simulink model "nonlinear_double_track_model"  (R2024b)
settings: Solver=VariableStepAuto  StartTime=0.0  StopTime=5  FixedStep=0.01  RelTol=1e-4
undefined symbols (not defined in the file or in its folder): a, b, balance_bar_ratio_front, brake_pedal_motion_ratio, cg_height, disc_radius_front, disc_radius_rear, front_mass_distribution, g, gear_ratio, Jeq, Ki_yaw, Kp_yaw, mass_total, max_pedal_force, mc_diameter_front, mc_diameter_rear, num_pistons_front, num_pistons_rear, pad_friction_front, pad_friction_rear, pad_height_front, pad_height_rear, piston_radius_front, piston_radius_rear, static_toe_front, static_toe_rear, tire_loaded_radius, tire_mu, tire_mu_correction_factor, tlltd, toe_deflection_rear, track_width_front, track_width_rear, wheelbase, yaw_polar_inertia
libraries: simulink_extras x12, checkpacklib x1, slpidlib x1
(part 6 of 6)

== matlab function nonlinear_double_track_model/Vehicle Plant Model/Slip Ratio Calculation/Slip Ratio Calculation ==
  data: sl_lf output; sl_rf output; sl_lr output; sl_rr output; w_fl input; w_fr input; w_rl input; w_rr input; vs_fl input; vs_fr input; vs_rl input; vs_rr input; effective_radius input
%{

Slip Ratio:
We should expect SL to be (+) when the wheel is spinning faster than the
tire surface velocity, resulting in forward acceleration. SL should be (-)
for drag or braking forces, resuling in negative long acceleration.

%}
function [sl_lf, sl_rf, sl_lr, sl_rr] = fcn(w_fl, w_fr, w_rl, w_rr, vs_fl, vs_fr, vs_rl, vs_rr, effective_radius)

% Note: in calspan info N is in RPM. Here we use rad/s so we compare
% apples-to-apples. No difference.

%% Calculate slip angles
% Note: a better approach is to calculate RE as a function of V, Fz ->
% RE(V, Fz) for each wheel individually, and use a lookup table for RE
% derived from the tire test data
sl_lf = (w_fl * effective_radius / vs_fl) - 1;
sl_rf = (w_fr * effective_radius / vs_fr) - 1;
sl_lr = (w_rl * effective_radius / vs_rl) - 1;
sl_rr = (w_rr * effective_radius / vs_rr) - 1;

== matlab function nonlinear_double_track_model/Vehicle Plant Model/Mechanical Brake System/Brake Torque FL ==
  data: pressure input; brake_torque output; wheel_speed input; piston_radius input; num_pistons input; pad_height input; mu input; disc_radius input
function brake_torque = fcn(pressure, wheel_speed, piston_radius, num_pistons, pad_height, mu, disc_radius)

% caculate radius to center of pad
pad_center_radius = disc_radius - (pad_height / 2);

% calculate total piston area per caliper
piston_area = pi * (piston_radius)^2 * num_pistons;

% calculate caliper force
caliper_force = mu * pressure * piston_area;

% calculate brake torque
if (wheel_speed > 0)
    brake_torque = -caliper_force * pad_center_radius;
elseif (wheel_speed < 0)
    brake_torque = caliper_force * pad_center_radius;
else
    % Note: for speed = 0, the proper brake torque is equal & opposite to
    % the applied wheel torque. to do some day, but i dont care
    brake_torque = 0;
end

== matlab function nonlinear_double_track_model/Vehicle Plant Model/Mechanical Brake System/Brake Torque FL2 ==
  data: pressure input; brake_torque output; wheel_speed input; piston_radius input; num_pistons input; pad_height input; mu input; disc_radius input
function brake_torque = fcn(pressure, wheel_speed, piston_radius, num_pistons, pad_height, mu, disc_radius)

% caculate radius to center of pad
pad_center_radius = disc_radius - (pad_height / 2);

% calculate total piston area per caliper
piston_area = pi * (piston_radius)^2 * num_pistons;

% calculate caliper force
caliper_force = mu * pressure * piston_area;

% calculate brake torque
if (wheel_speed > 0)
    brake_torque = -caliper_force * pad_center_radius;
elseif (wheel_speed < 0)
    brake_torque = caliper_force * pad_center_radius;
else
    % Note: for speed = 0, the proper brake torque is equal & opposite to
    % the applied wheel torque. to do some day, but i dont care
    brake_torque = 0;
end

== matlab function nonlinear_double_track_model/Vehicle Plant Model/Mechanical Brake System/Brake Torque FL3 ==
  data: pressure input; brake_torque output; wheel_speed input; piston_radius input; num_pistons input; pad_height input; mu input; disc_radius input
function brake_torque = fcn(pressure, wheel_speed, piston_radius, num_pistons, pad_height, mu, disc_radius)

% caculate radius to center of pad
pad_center_radius = disc_radius - (pad_height / 2);

% calculate total piston area per caliper
piston_area = pi * (piston_radius)^2 * num_pistons;

% calculate caliper force
caliper_force = mu * pressure * piston_area;

% calculate brake torque
if (wheel_speed > 0)
    brake_torque = -caliper_force * pad_center_radius;
elseif (wheel_speed < 0)
    brake_torque = caliper_force * pad_center_radius;
else
    % Note: for speed = 0, the proper brake torque is equal & opposite to
    % the applied wheel torque. to do some day, but i dont care
    brake_torque = 0;
end

== matlab function nonlinear_double_track_model/Vehicle Plant Model/Aero Forces/MATLAB Function ==
  data: a input; downforce_front output; b input; downforce input; center_of_pressure input; downforce_rear output
function [downforce_front, downforce_rear] = fcn(a, b, downforce, center_of_pressure)

downforce_front = downforce * (center_of_pressure + b) / (a + b);
downforce_rear = downforce * (a - center_of_pressure) / (a + b);

== matlab function nonlinear_double_track_model/Vehicle Plant Model/Wheel Dynamics/Initial Velocity to Initial wheel speed ==
  data: v input; w output; r input
function w = fcn(v, r)

w = v/r;

== matlab function nonlinear_double_track_model/Vehicle Plant Model/Normal Force Calculation1/Normal Load Calculation ==
  data: total_mass input; fz_fl output; fz_fr output; fz_rl output; fz_rr output; g input; front_mass_distribution input; tlltd input; cg_height input; track_width_front input; track_width_rear input; wheelbase input; fy_vehicle input; fx_vehicle input; fz_total output; on_two_wheels output; front_downforce input; rear_downforce input
%{
    
Calculates the normal forces on each tire. It considers the static normal
load, long. load transfer, lateral load transfer using a distribution
constant, and checks for tires lifiting off the ground. It ensures the
total normal load is equal to the vehicle normal force. When multiple tires
are off the ground the load distribution in the grounded tires may be off.
Load transfer from Mx is not implemented.

Note: for aerodynamics add those after this block and not in it.

Inputs:
- total_mass: Total mass of the vehicle (kg)
- g: Gravitational acceleration (m/s^2)
- static_wd: Static weight distribution as [front, rear]
- tlltd: Total lateral load transfer distribution (percentage)
- longitudinal_transfer: Longitudinal load transfer (N)
- lateral_transfer: Lateral load transfer (N)
- fy_vehicle: total lateral force acting on vehicle CG (N)
- fx_vehicle: total longitudinal force acting on vehicle CG (N)
- front_downforce: downforce at front axle. This will be negative (N)
- rear_downforce: downforce at rear axle. This will be negative (N)

Force Directions
when looking from th top of the vehicle:
(+) fx_vehicle is forward
(+) fy_vehicle is to the left

%}

function [fz_fl, fz_fr, fz_rl, fz_rr, fz_total, on_two_wheels] = normal_loads(total_mass, g, front_mass_distribution, tlltd, cg_height, track_width_front, track_width_rear, wheelbase, fy_vehicle, fx_vehicle, front_downforce, rear_downforce)

%% Constants and initial calculations
total_normal_load = total_mass * g; % Total vertical load (N)
static_load_front = front_mass_distribution * total_normal_load;
static_load_rear = (1 - front_mass_distribution) * total_normal_load;
track_width = (track_width_front + track_width_rear) / 2;

%% Static normal loads  as [FL, FR, RL, RR]
Fz_static = [static_load_front / 2, static_load_front / 2, static_load_rear / 2, static_load_rear / 2];

%% Calculate lateral load transfer
% from left tires to right tires
lateral_transfer = (fy_vehicle * cg_height) / track_width;
Fz_transfer_lat = lateral_transfer .* [-tlltd, tlltd, -(1 - tlltd), (1 - tlltd)];


%% Calculate longitudinal load transfer
% from front tires to rear tires
longitudinal_transfer = (fx_vehicle * cg_height) / wheelbase;
Fz_transfer_long = longitudinal_transfer .* [-1/2, -1/2, 1/2, 1/2];

%% Calculate downforce contribution
Fz_downforce = [-front_downforce/2, -front_downforce/2, -rear_downforce/2, -rear_downforce/2];
total_downforce = sum(Fz_downforce);

% Combine static and transfer loads
fz = Fz_static + Fz_transfer_long + Fz_transfer_lat + Fz_downforce;

%% Clamp negative loads to zero
fz(fz < 0) = 0; % if a tire is off the ground, make it zero so tire model doesnt go crazy

%% Check if redistribution is necessary
% find the tires that are on the ground
grounded_tires = fz > 0;

% Redistribution is needed only if a tire is off the ground
if sum(grounded_tires) < 4

    % correct the other tire normal loads for the contact adjustment
    % the excess load which can be distributed cannot be the static normal
    % load or the downforce - only from load transfer reactions
    excess_load = sum(fz) - total_normal_load - total_downforce; 
    
    % We will subtract load from tires that are contacting the ground based
    % on the ratios of their normal loads
    fz = fz - (excess_load .* (fz ./ (sum(fz(grounded_tires)))));
end

% invert for the tire model:
fz = - fz;

fz_total = sum(fz);

fz_fl = fz(1);
fz_fr = fz(2);
fz_rl = fz(3);
fz_rr = fz(4);

%% Output to check if the vehicle is up on two wheels

if (fz == 0)
    on_two_wheels = 1;
else
    on_two_wheels = 0;
end

== matlab function nonlinear_double_track_model/Reference Yaw Rate Generator/MATLAB Function ==
  data: velocity input; ref_yaw_rate output; steer_angles input; mu input; sigma input; wheelbase input; g input
function ref_yaw_rate = fcn(velocity, steer_angles, mu, sigma, wheelbase, g)

yaw_rate_max=sigma*mu*g/velocity;

% Calculate average front tire steer angle
delta = (steer_angles(1) + steer_angles(2)) / 2;

Ku=0; % target understeer gradient
yaw_rate=velocity/(wheelbase+Ku*velocity^2)*delta;

if(yaw_rate>yaw_rate_max)
    ref_yaw_rate=yaw_rate_max;
else
    ref_yaw_rate=yaw_rate;
end

== matlab function nonlinear_double_track_model/Vehicle Plant Model/Double Track Vehicle Dynamics/Body Frame Acceleration ==
  data: tire_forces input; steering_angles input; fx_total output; fy_total output; body_acceleration_x output; body_acceleration_y output; mass_total input; body_velocity_x input; body_velocity_y input; yaw_rate input; aero_drag input; zero_long_force input
%{

Solve the equation of motion for longitudianl acceleration

Sum(Fx) / mass = long_acceleration

%% Inputs:

Tire Forces [N]: tire_forces = [fy_fl, fy_fr, fy_rl, fy_rr; fx_fl, fx_fr, fx_rl, fx_rr]; 
Steering Angles [rad]: steering_angles = [delta_fl, delta_fr, delta_rl, delta_rr];
aero_drag [N]: expected in vehicle coordinate system, so it should be
negative

%% Outputs:

fx_total [N]
fy_total [N]
body_acceleration_x [m/s^2]
body_acceleration_y [m/s^2]

%}
function [fx_total, fy_total, body_acceleration_x, body_acceleration_y]  = fcn(tire_forces, steering_angles, mass_total, body_velocity_x, body_velocity_y, yaw_rate, aero_drag, zero_long_force)

%% convert input matricies into individual forces for code clarity
fy_fl = tire_forces(1, 1);
fy_fr = tire_forces(1, 2);
fy_rl = tire_forces(1, 3);
fy_rr = tire_forces(1, 4);

fx_fl = tire_forces(2, 1);
fx_fr = tire_forces(2, 2);
fx_rl = tire_forces(2, 3);
fx_rr = tire_forces(2, 4);

delta_fl = steering_angles(1);
delta_fr = steering_angles(2);
delta_rl = steering_angles(3);
delta_rr = steering_angles(4);

%% Convert tire forces into vehicle body frame
fx_body_fl = -fy_fl * sin(delta_fl) + fx_fl * cos(delta_fl);
fy_body_fl = fy_fl * cos(delta_fl) + fx_fl * sin(delta_fl);

fx_body_fr = -fy_fr * sin(delta_fr) + fx_fr * cos(delta_fr);
fy_body_fr = fy_fr * cos(delta_fr) + fx_fr * sin(delta_fr);

fx_body_rl = -fy_rl * sin(delta_rl) + fx_rl * cos(delta_rl);
fy_body_rl = fy_rl * cos(delta_rl) + fx_rl * sin(delta_rl);

fx_body_rr = -fy_rr * sin(delta_rr) + fx_rr * cos(delta_rr);
fy_body_rr = fy_rr * cos(delta_rr) + fx_rr * sin(delta_rr);

%% Calculate Longitudinal force and acceleration
if (zero_long_force)
    % enable this to approximate steady-state cornering 
    fx_total = -(yaw_rate * body_velocity_y) * mass_total; % this ensures x acceleration is always zero
else
    fx_total = fx_body_fl + fx_body_fr + fx_body_rl + fx_body_rr + aero_drag; % used for load transfer
end

body_acceleration_x = (fx_total / mass_total) + (yaw_rate * body_velocity_y); % must account for the apparent coriolis force

%% Calculate Lateral force and acceleration
fy_total = fy_body_fl + fy_body_fr + fy_body_rl + fy_body_rr; % used for load transfer
body_acceleration_y = fy_total / mass_total - (yaw_rate * body_velocity_x);  % must account for the apparent coriolis force

== matlab function nonlinear_double_track_model/Vehicle Plant Model/Double Track Vehicle Dynamics/Side-Slip Angle Calculation ==
  data: velocity_x input; beta output; velocity_y input
%{

Calculates the side-slip angle based on the angle produced by the lateral
and longitudinal velocities. Beta will vary from -pi to pi.

%}
function beta = fcn(velocity_x, velocity_y)

beta = atan(velocity_y / velocity_x);

== matlab function nonlinear_double_track_model/Vehicle Plant Model/Double Track Vehicle Dynamics/Body Frame to Fixed Inertial Frame ==
  data: yaw_angle input; velocity_x output; body_velocity_x input; body_velocity_y input; velocity_y output
%{
%% Double Track Vehicle Dynamics

%% Overview
These equations describe the vehicle dynamics of a double track vehicle
model for longitudinal & lateral directions, and in the yaw axis. The model
keeps track of a fixed inertial frame for vehicle position and orientation.
The vehicle forces and velocities are described in a body-fixed refernce
frame.

%}
function [velocity_x, velocity_y] = fcn(yaw_angle, body_velocity_x, body_velocity_y)

velocity_x = body_velocity_x * cos(yaw_angle) - body_velocity_y * sin(yaw_angle);
velocity_y = body_velocity_x * sin(yaw_angle) + body_velocity_y * cos(yaw_angle);

== matlab function nonlinear_double_track_model/Vehicle Plant Model/Double Track Vehicle Dynamics/Yaw Acceleration ==
  data: tire_forces input; yaw_moment output; tire_moments input; external_moment input; steering_angles input; yaw_inertia input; track_width_front input; track_width_rear input; wheelbase_front input; wheelbase_rear input; yaw_acceleration output
%{

Solve the equation of motion for acceleration around the yaw axis

Sum(M) / Izz = yaw_acceleration

%% Inputs:

Tire Forces [N]: tire_forces = [fy_fl, fy_fr, fy_rl, fy_rr; fx_fl, fx_fr, fx_rl, fx_rr]; 
Tire Moments [Nm]: tire_moments = [mz_fl, mz_fr, mz_rl, mz_rr];
external_moment [Nm]: external moment applied to vehicle CG position
Steering Angles [rad]: steering_angles = [delta_fl, delta_fr, delta_rl, delta_rr];

%% Outputs:

yaw_moment [Nm]
yaw_acceleration [rad/s^2]

%}
function [yaw_moment, yaw_acceleration]  = fcn(tire_forces, tire_moments, external_moment, steering_angles, yaw_inertia, track_width_front, track_width_rear, wheelbase_front, wheelbase_rear)

%% convert input matricies into individual forces for code clarity
fy_fl = tire_forces(1, 1);
fy_fr = tire_forces(1, 2);
fy_rl = tire_forces(1, 3);
fy_rr = tire_forces(1, 4);

fx_fl = tire_forces(2, 1);
fx_fr = tire_forces(2, 2);
fx_rl = tire_forces(2, 3);
fx_rr = tire_forces(2, 4);

mz_fl = tire_moments(1);
mz_fr = tire_moments(2);
mz_rl = tire_moments(3);
mz_rr = tire_moments(4);

delta_fl = steering_angles(1);
delta_fr = steering_angles(2);
delta_rl = steering_angles(3);
delta_rr = steering_angles(4);


%% Convert tire forces into vehicle body frame
fx_body_fl = -fy_fl * sin(delta_fl) + fx_fl * cos(delta_fl);
fy_body_fl = fy_fl * cos(delta_fl) + fx_fl * sin(delta_fl);

fx_body_fr = -fy_fr * sin(delta_fr) + fx_fr * cos(delta_fr);
fy_body_fr = fy_fr * cos(delta_fr) + fx_fr * sin(delta_fr);

fx_body_rl = -fy_rl * sin(delta_rl) + fx_rl * cos(delta_rl);
fy_body_rl = fy_rl * cos(delta_rl) + fx_rl * sin(delta_rl);

fx_body_rr = -fy_rr * sin(delta_rr) + fx_rr * cos(delta_rr);
fy_body_rr = fy_rr * cos(delta_rr) + fx_rr * sin(delta_rr);

%% Calculate moment contributions
m_fl = -fx_body_fl * track_width_front / 2 + fy_body_fl * wheelbase_front;
m_fr = fx_body_fr * track_width_front / 2 + fy_body_fr * wheelbase_front;
m_rl = -fx_body_rl * track_width_rear / 2 - fy_body_rl * wheelbase_rear;
m_rr = fx_body_rr * track_width_rear / 2 - fy_body_rr * wheelbase_rear;

%% Calculate total yaw moment & acceleration
yaw_moment = (m_fl + m_fr + m_rl + m_rr) + (mz_fl + mz_fr + mz_rl + mz_rr) + external_moment; % sum moments from tire forces and self-aligning moments
yaw_acceleration = yaw_moment / yaw_inertia;

== matlab function nonlinear_double_track_model/Vehicle Plant Model/Wheel Dynamics/T_load1 ==
  data: Fx input; gear_ratio input; T_load output; wheel_radius input
function T_load = fcn(Fx, gear_ratio, wheel_radius)

T_load=wheel_radius/gear_ratio*Fx; % 'load torque' calculation

== matlab function nonlinear_double_track_model/Vehicle Plant Model/Wheel Dynamics/T_load2 ==
  data: Fx input; gear_ratio input; T_load output; wheel_radius input
function T_load = fcn(Fx, gear_ratio, wheel_radius)

T_load=wheel_radius/gear_ratio*Fx; % 'load torque' calculation

== matlab function nonlinear_double_track_model/Vehicle Plant Model/Wheel Dynamics/T_load3 ==
  data: Fx input; gear_ratio input; T_load output; wheel_radius input
function T_load = fcn(Fx, gear_ratio, wheel_radius)

T_load=wheel_radius/gear_ratio*Fx; % 'load torque' calculation

== matlab function nonlinear_double_track_model/Vehicle Plant Model/Wheel Dynamics/T_load4 ==
  data: Fx input; gear_ratio input; T_load output; wheel_radius input
function T_load = fcn(Fx, gear_ratio, wheel_radius)

T_load=wheel_radius/gear_ratio*Fx; % 'load torque' calculation

== matlab function nonlinear_double_track_model/Vehicle Plant Model/Slip Angle Calculation/Slip Angle Calculation ==
  data: y_dot input; alpha_fl output; x_dot input; r input; delta_fl input; delta_fr input; delta_rl input; delta_rr input; a input; b input; alpha_fr output; alpha_rl output; alpha_rr output; tf input; tr input
%{

%% Overview:
This block computes the slip angle in radians for each tire.

y_dot: vehicle cg velocity along vehicle y axis (m/s)
x_dot: vehicle cg velocity along vehicle x axis (m/s)

r: vehicle yaw rate (rad/s)

delta_fl: front left wheel steering angle (rad)
delta_fr: front right wheel steering angle (rad)
delta_rl: rear left wheel steering angle (rad)
delta_rr: rear right wheel steering angle (rad)

a: distance of front wheels to cg viewed from the top
b: distance of rear wheels to cg viewed from the top

tf: front track width
tr: rear track width

alpha_fl: front wheel slip angle
alpha_fr: front wheel slip angle
alpha_rl: rear wheel slip angle
alpha_rr: rear wheel slip angle

%% Coordinate systems
- using ISO 8855 vehicle coordinate system (Vy is pointing to the left)
- using SAE tire coordinate system (where when the tire velocity direction
is to the right of the tire heading, the slip angle is positive)

%}

function [alpha_fl, alpha_fr, alpha_rl, alpha_rr] = fcn(y_dot, x_dot, r, delta_fl, delta_fr, delta_rl, delta_rr, a, b, tf, tr)

alpha_fl = delta_fl - atan((y_dot + (a * r))/(x_dot - (r * tf/2)));
alpha_fr = delta_fr - atan((y_dot + (a * r))/(x_dot + (r * tf/2)));
alpha_rl = delta_rl - atan((y_dot - (b * r))/(x_dot - (r * tr/2)));
alpha_rr = delta_rr - atan((y_dot - (b * r))/(x_dot + (r * tr/2)));

== matlab function nonlinear_double_track_model/Vehicle Plant Model/Mechanical Brake System/Brake Pressure Calculation ==
  data: pedal_percentage input; front_pressure output; pedal_max_force input; motion_ratio input; balance_bar_ratio_front input; rear_pressure output; mc_diameter_front input; mc_diameter_rear input
function [front_pressure, rear_pressure] = fcn(pedal_percentage, pedal_max_force, motion_ratio, balance_bar_ratio_front, mc_diameter_front, mc_diameter_rear)

% Calculate pedal force
pedal_force = pedal_percentage * pedal_max_force;

% Calculate master cylinder forces
balance_bar_force = pedal_force * motion_ratio;
mc_force_front = balance_bar_force * balance_bar_ratio_front;
mc_force_rear = balance_bar_force * (1 - balance_bar_ratio_front);

% Calculate brake line pressures
front_pressure = mc_force_front / (pi * (mc_diameter_front/2)^2);  % N/m
rear_pressure = mc_force_rear / (pi * (mc_diameter_rear/2)^2);

== matlab function nonlinear_double_track_model/Vehicle Plant Model/Mechanical Brake System/Brake Torque FL1 ==
  data: pressure input; brake_torque output; wheel_speed input; piston_radius input; num_pistons input; pad_height input; mu input; disc_radius input
function brake_torque = fcn(pressure, wheel_speed, piston_radius, num_pistons, pad_height, mu, disc_radius)

% caculate radius to center of pad
pad_center_radius = disc_radius - (pad_height / 2);

% calculate total piston area per caliper
piston_area = pi * (piston_radius)^2 * num_pistons;

% calculate caliper force
caliper_force = mu * pressure * piston_area;

% calculate brake torque
if (wheel_speed > 0)
    brake_torque = -caliper_force * pad_center_radius;
elseif (wheel_speed < 0)
    brake_torque = caliper_force * pad_center_radius;
else
    % Note: for speed = 0, the proper brake torque is equal & opposite to
    % the applied wheel torque. to do some day, but i dont care
    brake_torque = 0;
end

== matlab function nonlinear_double_track_model/Vehicle Plant Model/Toe Compliance Model/Rear Toe Compliance from Fy ==
  data: eyr_gain input; fy_rl input; toe_change_rl output; fy_rr input; toe_change_rr output
%{

Calculates rear toe change due to rear toe compliance. See compliance
models.

%}
function [toe_change_rl, toe_change_rr] = fcn(eyr_gain, fy_rl, fy_rr)

toe_change_rl = fy_rl * (eyr_gain / 1000);
toe_change_rr = fy_rr * (eyr_gain / 1000);

== matlab function nonlinear_double_track_model/Vehicle Plant Model/Slip Ratio Calculation/Tire Surface Velocity Calculation ==
  data: body_velocity_x input; vs_fl output; body_velocity_y input; yaw_rate input; delta_fl input; delta_fr input; delta_rl input; delta_rr input; alpha_fl input; alpha_fr input; alpha_rl input; alpha_rr input; track_width_front input; track_width_rear input; a input; b input; vs_fr output; vs_rl output; vs_rr output
function [vs_fl, vs_fr, vs_rl, vs_rr] = fcn(body_velocity_x, body_velocity_y, yaw_rate, delta_fl, delta_fr, delta_rl, delta_rr, alpha_fl, alpha_fr, alpha_rl, alpha_rr, track_width_front, track_width_rear, a, b)

vs_fl = (body_velocity_x - yaw_rate * track_width_front / 2) * cos(delta_fl - alpha_fl) + (body_velocity_y + yaw_rate * a) * sin(delta_fl - alpha_fl);
vs_fr = (body_velocity_x + yaw_rate * track_width_front / 2) * cos(delta_fr - alpha_fr) + (body_velocity_y + yaw_rate * a) * sin(delta_fr - alpha_fr);
vs_rl = (body_velocity_x - yaw_rate * track_width_rear / 2) * cos(delta_rl - alpha_rl) + (body_velocity_y - yaw_rate * b) * sin(delta_rl - alpha_rl);
vs_rr = (body_velocity_x + yaw_rate * track_width_rear / 2) * cos(delta_rr - alpha_rr) + (body_velocity_y - yaw_rate * b) * sin(delta_rr - alpha_rr);
